simulink model "os_polyphase_synth_4096c"  (R2021a) (continued)
== system os_polyphase_synth_4096c/pfb_fir/pol1_in1_tap80 == (continued)
      | (D-ACIN)*(D-ACIN)+PCIN>>17+C+CARRYIN
      | (D-ACIN)*(D-ACIN)+PCIN>>17+CARRYIN
      | (D-ACIN)*(D-ACIN)+PCIN>>17+P
      | (D-ACIN)*(D-ACIN)+PCIN>>17+P+CARRYIN
      | (D-ACIN)*(D-ACIN)-C
      | (D-ACIN)*(D-ACIN)-P
      | (D-ACIN)*(D-ACIN)-P>>17
      | (D-ACIN)*(D-ACIN)-PCIN
      | (D-ACIN)*(D-ACIN)-PCIN>>17
      | (D-ACIN)*B
      | (D-ACIN)*B+C
      | (D-ACIN)*B+C+C
      | (D-ACIN)*B+C+C+CARRYIN
      | (D-ACIN)*B+C+CARRYIN
      | (D-ACIN)*B+C+P
      | (D-ACIN)*B+C+P+CARRYIN
      | (D-ACIN)*B+C+P>>17
      | (D-ACIN)*B+C+P>>17+CARRYIN
      | (D-ACIN)*B+C+PCIN
      | (D-ACIN)*B+C+PCIN+CARRYIN
      | (D-ACIN)*B+C+PCIN>>17
      | (D-ACIN)*B+C+PCIN>>17+CARRYIN
      | (D-ACIN)*B+C-C
      | (D-ACIN)*B+C-P
      | (D-ACIN)*B+C-P>>17
      | (D-ACIN)*B+C-PCIN
      | (D-ACIN)*B+C-PCIN>>17
      | (D-ACIN)*B+CARRYIN
      | (D-ACIN)*B+P
      | (D-ACIN)*B+P+C
      | (D-ACIN)*B+P+C+CARRYIN
      | (D-ACIN)*B+P+CARRYIN
      | (D-ACIN)*B+P+P
      | (D-ACIN)*B+P+P+CARRYIN
      | (D-ACIN)*B+P+P>>17
      | (D-ACIN)*B+P+P>>17+CARRYIN
      | (D-ACIN)*B+P+PCIN
      | (D-ACIN)*B+P+PCIN+CARRYIN
      | (D-ACIN)*B+P+PCIN>>17
      | (D-ACIN)*B+P+PCIN>>17+CARRYIN
      | (D-ACIN)*B+P-C
      | (D-ACIN)*B+P-P
      | (D-ACIN)*B+P-P>>17
      | (D-ACIN)*B+P-PCIN
      | (D-ACIN)*B+P-PCIN>>17
      | (D-ACIN)*B+P>>17
      | (D-ACIN)*B+P>>17+C
      | (D-ACIN)*B+P>>17+C+CARRYIN
      | (D-ACIN)*B+P>>17+CARRYIN
      | (D-ACIN)*B+P>>17+P
      | (D-ACIN)*B+P>>17+P+CARRYIN
      | (D-ACIN)*B+PCIN
      | (D-ACIN)*B+PCIN+C
      | (D-ACIN)*B+PCIN+C+CARRYIN
      | (D-ACIN)*B+PCIN+CARRYIN
      | (D-ACIN)*B+PCIN+P
      | (D-ACIN)*B+PCIN+P+CARRYIN
      | (D-ACIN)*B+PCIN>>17
      | (D-ACIN)*B+PCIN>>17+C
      | (D-ACIN)*B+PCIN>>17+C+CARRYIN
      | (D-ACIN)*B+PCIN>>17+CARRYIN
      | (D-ACIN)*B+PCIN>>17+P
      | (D-ACIN)*B+PCIN>>17+P+CARRYIN
      | (D-ACIN)*B-C
      | (D-ACIN)*B-P
      | (D-ACIN)*B-P>>17
      | (D-ACIN)*B-PCIN
      | (D-ACIN)*B-PCIN>>17
      | (D-ACIN)*BCIN
      | (D-ACIN)*BCIN+C
      | (D-ACIN)*BCIN+C+C
      | (D-ACIN)*BCIN+C+C+CARRYIN
      | (D-ACIN)*BCIN+C+CARRYIN
      | (D-ACIN)*BCIN+C+P
      | (D-ACIN)*BCIN+C+P+CARRYIN
      | (D-ACIN)*BCIN+C+P>>17
      | (D-ACIN)*BCIN+C+P>>17+CARRYIN
      | (D-ACIN)*BCIN+C+PCIN
      | (D-ACIN)*BCIN+C+PCIN+CARRYIN
      | (D-ACIN)*BCIN+C+PCIN>>17
      | (D-ACIN)*BCIN+C+PCIN>>17+CARRYIN
      | (D-ACIN)*BCIN+C-C
      | (D-ACIN)*BCIN+C-P
      | (D-ACIN)*BCIN+C-P>>17
      | (D-ACIN)*BCIN+C-PCIN
      | (D-ACIN)*BCIN+C-PCIN>>17
      | (D-ACIN)*BCIN+CARRYIN
      | (D-ACIN)*BCIN+P
      | (D-ACIN)*BCIN+P+C
      | (D-ACIN)*BCIN+P+C+CARRYIN
      | (D-ACIN)*BCIN+P+CARRYIN
      | (D-ACIN)*BCIN+P+P
      | (D-ACIN)*BCIN+P+P+CARRYIN
      | (D-ACIN)*BCIN+P+P>>17
      | (D-ACIN)*BCIN+P+P>>17+CARRYIN
      | (D-ACIN)*BCIN+P+PCIN
      | (D-ACIN)*BCIN+P+PCIN+CARRYIN
      | (D-ACIN)*BCIN+P+PCIN>>17
      | (D-ACIN)*BCIN+P+PCIN>>17+CARRYIN
      | (D-ACIN)*BCIN+P-C
      | (D-ACIN)*BCIN+P-P
      | (D-ACIN)*BCIN+P-P>>17
      | (D-ACIN)*BCIN+P-PCIN
      | (D-ACIN)*BCIN+P-PCIN>>17
      | (D-ACIN)*BCIN+P>>17
      | (D-ACIN)*BCIN+P>>17+C
      | (D-ACIN)*BCIN+P>>17+C+CARRYIN
      | (D-ACIN)*BCIN+P>>17+CARRYIN
      | (D-ACIN)*BCIN+P>>17+P
      | (D-ACIN)*BCIN+P>>17+P+CARRYIN
      | (D-ACIN)*BCIN+PCIN
      | (D-ACIN)*BCIN+PCIN+C
      | (D-ACIN)*BCIN+PCIN+C+CARRYIN
      | (D-ACIN)*BCIN+PCIN+CARRYIN
      | (D-ACIN)*BCIN+PCIN+P
      | (D-ACIN)*BCIN+PCIN+P+CARRYIN
      | (D-ACIN)*BCIN+PCIN>>17
      | (D-ACIN)*BCIN+PCIN>>17+C
      | (D-ACIN)*BCIN+PCIN>>17+C+CARRYIN
      | (D-ACIN)*BCIN+PCIN>>17+CARRYIN
      | (D-ACIN)*BCIN+PCIN>>17+P
      | (D-ACIN)*BCIN+PCIN>>17+P+CARRYIN
      | (D-ACIN)*BCIN-C
      | (D-ACIN)*BCIN-P
      | (D-ACIN)*BCIN-P>>17
      | (D-ACIN)*BCIN-PCIN
      | (D-ACIN)*BCIN-PCIN>>17
      | (D-ACIN)+C
      | (D-ACIN)+C+C
      | (D-ACIN)+C+C+CARRYIN
      | (D-ACIN)+C+CARRYIN
      | (D-ACIN)+C+P
      | (D-ACIN)+C+P+CARRYIN
      | (D-ACIN)+C+P>>17
      | (D-ACIN)+C+P>>17+CARRYIN
      | (D-ACIN)+C+PCIN
      | (D-ACIN)+C+PCIN+CARRYIN
      | (D-ACIN)+C+PCIN>>17
      | (D-ACIN)+C+PCIN>>17+CARRYIN
      | (D-ACIN)+C-C
      | (D-ACIN)+C-P
      | (D-ACIN)+C-P>>17
      | (D-ACIN)+C-PCIN
      | (D-ACIN)+C-PCIN>>17
      | (D-ACIN)+CARRYIN
      | (D-ACIN)+P
      | (D-ACIN)+P+C
      | (D-ACIN)+P+C+CARRYIN
      | (D-ACIN)+P+CARRYIN
      | (D-ACIN)+P+P
      | (D-ACIN)+P+P+CARRYIN
      | (D-ACIN)+P+P>>17
      | (D-ACIN)+P+P>>17+CARRYIN
      | (D-ACIN)+P+PCIN
      | (D-ACIN)+P+PCIN+CARRYIN
      | (D-ACIN)+P+PCIN>>17
      | (D-ACIN)+P+PCIN>>17+CARRYIN
      | (D-ACIN)+P-C
      | (D-ACIN)+P-P
      | (D-ACIN)+P-P>>17
      | (D-ACIN)+P-PCIN
      | (D-ACIN)+P-PCIN>>17
      | (D-ACIN)+P>>17
      | (D-ACIN)+P>>17+C
      | (D-ACIN)+P>>17+C+CARRYIN
      | (D-ACIN)+P>>17+CARRYIN
      | (D-ACIN)+P>>17+P
      | (D-ACIN)+P>>17+P+CARRYIN
      | (D-ACIN)+PCIN
      | (D-ACIN)+PCIN+C
      | (D-ACIN)+PCIN+C+CARRYIN
      | (D-ACIN)+PCIN+CARRYIN
      | (D-ACIN)+PCIN+P
      | (D-ACIN)+PCIN+P+CARRYIN
      | (D-ACIN)+PCIN>>17
      | (D-ACIN)+PCIN>>17+C
      | (D-ACIN)+PCIN>>17+C+CARRYIN
      | (D-ACIN)+PCIN>>17+CARRYIN
      | (D-ACIN)+PCIN>>17+P
      | (D-ACIN)+PCIN>>17+P+CARRYIN
      | (D-ACIN)-C
      | (D-ACIN)-P
      | (D-ACIN)-P>>17
      | (D-ACIN)-PCIN
      | (D-ACIN)-PCIN>>17
      | -((A+D))
      | -((A+D)*(A+D))
      | -((A+D)*(A+D)+C)
      | -((A+D)*(A+D)+C+CARRYIN)
      | -((A+D)*(A+D)+CARRYIN)
      | -((A+D)*(A+D)+P)
      | -((A+D)*(A+D)+P+CARRYIN)
      | -((A+D)*B)
      | -((A+D)*B+C)
      | -((A+D)*B+C+CARRYIN)
      | -((A+D)*B+CARRYIN)
      | -((A+D)*B+P)
      | -((A+D)*B+P+CARRYIN)
      | -((A+D)*BCIN)
      | -((A+D)*BCIN+C)
      | -((A+D)*BCIN+C+CARRYIN)
      | -((A+D)*BCIN+CARRYIN)
      | -((A+D)*BCIN+P)
      | -((A+D)*BCIN+P+CARRYIN)
      | -((A+D)+C)
      | -((A+D)+C+CARRYIN)
      | -((A+D)+CARRYIN)
      | -((A+D)+P)
      | -((A+D)+P+CARRYIN)
      | -((ACIN+D))
      | -((ACIN+D)*(ACIN+D))
      | -((ACIN+D)*(ACIN+D)+C)
      | -((ACIN+D)*(ACIN+D)+C+CARRYIN)
      | -((ACIN+D)*(ACIN+D)+CARRYIN)
      | -((ACIN+D)*(ACIN+D)+P)
      | -((ACIN+D)*(ACIN+D)+P+CARRYIN)
      | -((ACIN+D)*B)
      | -((ACIN+D)*B+C)
      | -((ACIN+D)*B+C+CARRYIN)
      | -((ACIN+D)*B+CARRYIN)
      | -((ACIN+D)*B+P)
      | -((ACIN+D)*B+P+CARRYIN)
      | -((ACIN+D)*BCIN)
      | -((ACIN+D)*BCIN+C)
      | -((ACIN+D)*BCIN+C+CARRYIN)
      | -((ACIN+D)*BCIN+CARRYIN)
      | -((ACIN+D)*BCIN+P)
      | -((ACIN+D)*BCIN+P+CARRYIN)
      | -((ACIN+D)+C)
      | -((ACIN+D)+C+CARRYIN)
      | -((ACIN+D)+CARRYIN)
      | -((ACIN+D)+P)
      | -((ACIN+D)+P+CARRYIN)
      | -((D+A))
      | -((D+A)*(D+A))
      | -((D+A)*(D+A)+C)
      | -((D+A)*(D+A)+C+CARRYIN)
      | -((D+A)*(D+A)+CARRYIN)
      | -((D+A)*(D+A)+P)
      | -((D+A)*(D+A)+P+CARRYIN)
      | -((D+A)*B)
      | -((D+A)*B+C)
      | -((D+A)*B+C+CARRYIN)
      | -((D+A)*B+CARRYIN)
      | -((D+A)*B+P)
      | -((D+A)*B+P+CARRYIN)
      | -((D+A)*BCIN)
      | -((D+A)*BCIN+C)
      | -((D+A)*BCIN+C+CARRYIN)
      | -((D+A)*BCIN+CARRYIN)
      | -((D+A)*BCIN+P)
      | -((D+A)*BCIN+P+CARRYIN)
      | -((D+A)+C)
      | -((D+A)+C+CARRYIN)
      | -((D+A)+CARRYIN)
      | -((D+A)+P)
      | -((D+A)+P+CARRYIN)
      | -((D+ACIN))
      | -((D+ACIN)*(D+ACIN))
      | -((D+ACIN)*(D+ACIN)+C)
      | -((D+ACIN)*(D+ACIN)+C+CARRYIN)
      | -((D+ACIN)*(D+ACIN)+CARRYIN)
      | -((D+ACIN)*(D+ACIN)+P)
      | -((D+ACIN)*(D+ACIN)+P+CARRYIN)
      | -((D+ACIN)*B)
      | -((D+ACIN)*B+C)
      | -((D+ACIN)*B+C+CARRYIN)
      | -((D+ACIN)*B+CARRYIN)
      | -((D+ACIN)*B+P)
      | -((D+ACIN)*B+P+CARRYIN)
      | -((D+ACIN)*BCIN)
      | -((D+ACIN)*BCIN+C)
      | -((D+ACIN)*BCIN+C+CARRYIN)
      | -((D+ACIN)*BCIN+CARRYIN)
      | -((D+ACIN)*BCIN+P)
      | -((D+ACIN)*BCIN+P+CARRYIN)
      | -((D+ACIN)+C)
      | -((D+ACIN)+C+CARRYIN)
      | -((D+ACIN)+CARRYIN)
      | -((D+ACIN)+P)
      | -((D+ACIN)+P+CARRYIN)
      | -((D-A))
      | -((D-A)*(D-A))
      | -((D-A)*(D-A)+C)
      | -((D-A)*(D-A)+C+CARRYIN)
      | -((D-A)*(D-A)+CARRYIN)
      | -((D-A)*(D-A)+P)
      | -((D-A)*(D-A)+P+CARRYIN)
      | -((D-A)*B)
      | -((D-A)*B+C)
      | -((D-A)*B+C+CARRYIN)
      | -((D-A)*B+CARRYIN)
      | -((D-A)*B+P)
      | -((D-A)*B+P+CARRYIN)
      | -((D-A)*BCIN)
      | -((D-A)*BCIN+C)
      | -((D-A)*BCIN+C+CARRYIN)
      | -((D-A)*BCIN+CARRYIN)
      | -((D-A)*BCIN+P)
      | -((D-A)*BCIN+P+CARRYIN)
      | -((D-A)+C)
      | -((D-A)+C+CARRYIN)
      | -((D-A)+CARRYIN)
      | -((D-A)+P)
      | -((D-A)+P+CARRYIN)
      | -((D-ACIN))
      | -((D-ACIN)*(D-ACIN))
      | -((D-ACIN)*(D-ACIN)+C)
      | -((D-ACIN)*(D-ACIN)+C+CARRYIN)
      | -((D-ACIN)*(D-ACIN)+CARRYIN)
      | -((D-ACIN)*(D-ACIN)+P)
      | -((D-ACIN)*(D-ACIN)+P+CARRYIN)
      | -((D-ACIN)*B)
      | -((D-ACIN)*B+C)
      | -((D-ACIN)*B+C+CARRYIN)
      | -((D-ACIN)*B+CARRYIN)
      | -((D-ACIN)*B+P)
      | -((D-ACIN)*B+P+CARRYIN)
      | -((D-ACIN)*BCIN)
      | -((D-ACIN)*BCIN+C)
      | -((D-ACIN)*BCIN+C+CARRYIN)
      | -((D-ACIN)*BCIN+CARRYIN)
      | -((D-ACIN)*BCIN+P)
      | -((D-ACIN)*BCIN+P+CARRYIN)
      | -((D-ACIN)+C)
      | -((D-ACIN)+C+CARRYIN)
      | -((D-ACIN)+CARRYIN)
      | -((D-ACIN)+P)
      | -((D-ACIN)+P+CARRYIN)
      | -(A)
      | -(A*A)
      | -(A*A+C)
      | -(A*A+C+CARRYIN)
      | -(A*A+CARRYIN)
      | -(A*A+P)
      | -(A*A+P+CARRYIN)
      | -(A*B)
      | -(A*B+C)
      | -(A*B+C+CARRYIN)
      | -(A*B+CARRYIN)
      | -(A*B+P)
      | -(A*B+P+CARRYIN)
      | -(A*BCIN)
      | -(A*BCIN+C)
      | -(A*BCIN+C+CARRYIN)
      | -(A*BCIN+CARRYIN)
      | -(A*BCIN+P)
      | -(A*BCIN+P+CARRYIN)
      | -(A+C)
      | -(A+C+CARRYIN)
      | -(A+CARRYIN)
      | -(A+D)
      | -(A+D)*(A+D)
      | -(A+D)*(A+D)-C
      | -(A+D)*(A+D)-C-CARRYIN
      | -(A+D)*(A+D)-CARRYIN
      | -(A+D)*(A+D)-P
      | -(A+D)*(A+D)-P-CARRYIN
      | -(A+D)*B
      | -(A+D)*B-C
      | -(A+D)*B-C-CARRYIN
      | -(A+D)*B-CARRYIN
      | -(A+D)*B-P
      | -(A+D)*B-P-CARRYIN
      | -(A+D)*BCIN
      | -(A+D)*BCIN-C
      | -(A+D)*BCIN-C-CARRYIN
      | -(A+D)*BCIN-CARRYIN
      | -(A+D)*BCIN-P
      | -(A+D)*BCIN-P-CARRYIN
      | -(A+D)-C
      | -(A+D)-C-CARRYIN
      | -(A+D)-CARRYIN
      | -(A+D)-P
      | -(A+D)-P-CARRYIN
      | -(A+P)
      | -(A+P+CARRYIN)
      | -(ACIN)
      | -(ACIN*ACIN)
      | -(ACIN*ACIN+C)
      | -(ACIN*ACIN+C+CARRYIN)
      | -(ACIN*ACIN+CARRYIN)
      | -(ACIN*ACIN+P)
      | -(ACIN*ACIN+P+CARRYIN)
      | -(ACIN*B)
      | -(ACIN*B+C)
      | -(ACIN*B+C+CARRYIN)
      | -(ACIN*B+CARRYIN)
      | -(ACIN*B+P)
      | -(ACIN*B+P+CARRYIN)
      | -(ACIN*BCIN)
      | -(ACIN*BCIN+C)
      | -(ACIN*BCIN+C+CARRYIN)
      | -(ACIN*BCIN+CARRYIN)
      | -(ACIN*BCIN+P)
      | -(ACIN*BCIN+P+CARRYIN)
      | -(ACIN+C)
      | -(ACIN+C+CARRYIN)
      | -(ACIN+CARRYIN)
      | -(ACIN+D)
      | -(ACIN+D)*(ACIN+D)
      | -(ACIN+D)*(ACIN+D)-C
      | -(ACIN+D)*(ACIN+D)-C-CARRYIN
      | -(ACIN+D)*(ACIN+D)-CARRYIN
      | -(ACIN+D)*(ACIN+D)-P
      | -(ACIN+D)*(ACIN+D)-P-CARRYIN
      | -(ACIN+D)*B
      | -(ACIN+D)*B-C
      | -(ACIN+D)*B-C-CARRYIN
      | -(ACIN+D)*B-CARRYIN
      | -(ACIN+D)*B-P
      | -(ACIN+D)*B-P-CARRYIN
      | -(ACIN+D)*BCIN
      | -(ACIN+D)*BCIN-C
      | -(ACIN+D)*BCIN-C-CARRYIN
      | -(ACIN+D)*BCIN-CARRYIN
      | -(ACIN+D)*BCIN-P
      | -(ACIN+D)*BCIN-P-CARRYIN
      | -(ACIN+D)-C
      | -(ACIN+D)-C-CARRYIN
      | -(ACIN+D)-CARRYIN
      | -(ACIN+D)-P
      | -(ACIN+D)-P-CARRYIN
      | -(ACIN+P)
      | -(ACIN+P+CARRYIN)
      | -(B*(A+D))
      | -(B*(A+D)+C)
      | -(B*(A+D)+C+CARRYIN)
      | -(B*(A+D)+CARRYIN)
      | -(B*(A+D)+P)
      | -(B*(A+D)+P+CARRYIN)
      | -(B*(ACIN+D))
      | -(B*(ACIN+D)+C)
      | -(B*(ACIN+D)+C+CARRYIN)
      | -(B*(ACIN+D)+CARRYIN)
      | -(B*(ACIN+D)+P)
      | -(B*(ACIN+D)+P+CARRYIN)
      | -(B*(D+A))
      | -(B*(D+A)+C)
      | -(B*(D+A)+C+CARRYIN)
      | -(B*(D+A)+CARRYIN)
      | -(B*(D+A)+P)
      | -(B*(D+A)+P+CARRYIN)
      | -(B*(D+ACIN))
      | -(B*(D+ACIN)+C)
      | -(B*(D+ACIN)+C+CARRYIN)
      | -(B*(D+ACIN)+CARRYIN)
      | -(B*(D+ACIN)+P)
      | -(B*(D+ACIN)+P+CARRYIN)
      | -(B*(D-A))
      | -(B*(D-A)+C)
      | -(B*(D-A)+C+CARRYIN)
      | -(B*(D-A)+CARRYIN)
      | -(B*(D-A)+P)
      | -(B*(D-A)+P+CARRYIN)
      | -(B*(D-ACIN))
      | -(B*(D-ACIN)+C)
      | -(B*(D-ACIN)+C+CARRYIN)
      | -(B*(D-ACIN)+CARRYIN)
      | -(B*(D-ACIN)+P)
      | -(B*(D-ACIN)+P+CARRYIN)
      | -(B*A)
      | -(B*A+C)
      | -(B*A+C+CARRYIN)
      | -(B*A+CARRYIN)
      | -(B*A+P)
      | -(B*A+P+CARRYIN)
      | -(B*ACIN)
      | -(B*ACIN+C)
      | -(B*ACIN+C+CARRYIN)
      | -(B*ACIN+CARRYIN)
      | -(B*ACIN+P)
      | -(B*ACIN+P+CARRYIN)
      | -(B*D)
      | -(B*D+C)
      | -(B*D+C+CARRYIN)
      | -(B*D+CARRYIN)
      | -(B*D+P)
      | -(B*D+P+CARRYIN)
      | -(BCIN*(A+D))
      | -(BCIN*(A+D)+C)
      | -(BCIN*(A+D)+C+CARRYIN)
      | -(BCIN*(A+D)+CARRYIN)
      | -(BCIN*(A+D)+P)
      | -(BCIN*(A+D)+P+CARRYIN)
      | -(BCIN*(ACIN+D))
      | -(BCIN*(ACIN+D)+C)
      | -(BCIN*(ACIN+D)+C+CARRYIN)
      | -(BCIN*(ACIN+D)+CARRYIN)
      | -(BCIN*(ACIN+D)+P)
      | -(BCIN*(ACIN+D)+P+CARRYIN)
      | -(BCIN*(D+A))
      | -(BCIN*(D+A)+C)
      | -(BCIN*(D+A)+C+CARRYIN)
      | -(BCIN*(D+A)+CARRYIN)
      | -(BCIN*(D+A)+P)
      | -(BCIN*(D+A)+P+CARRYIN)
      | -(BCIN*(D+ACIN))
      | -(BCIN*(D+ACIN)+C)
      | -(BCIN*(D+ACIN)+C+CARRYIN)
      | -(BCIN*(D+ACIN)+CARRYIN)
      | -(BCIN*(D+ACIN)+P)
      | -(BCIN*(D+ACIN)+P+CARRYIN)
      | -(BCIN*(D-A))
      | -(BCIN*(D-A)+C)
      | -(BCIN*(D-A)+C+CARRYIN)
      | -(BCIN*(D-A)+CARRYIN)
      | -(BCIN*(D-A)+P)
      | -(BCIN*(D-A)+P+CARRYIN)
      | -(BCIN*(D-ACIN))
      | -(BCIN*(D-ACIN)+C)
      | -(BCIN*(D-ACIN)+C+CARRYIN)
      | -(BCIN*(D-ACIN)+CARRYIN)
      | -(BCIN*(D-ACIN)+P)
      | -(BCIN*(D-ACIN)+P+CARRYIN)
      | -(BCIN*A)
      | -(BCIN*A+C)
      | -(BCIN*A+C+CARRYIN)
      | -(BCIN*A+CARRYIN)
      | -(BCIN*A+P)
      | -(BCIN*A+P+CARRYIN)
      | -(BCIN*ACIN)
      | -(BCIN*ACIN+C)
      | -(BCIN*ACIN+C+CARRYIN)
      | -(BCIN*ACIN+CARRYIN)
      | -(BCIN*ACIN+P)
      | -(BCIN*ACIN+P+CARRYIN)
      | -(BCIN*D)
      | -(BCIN*D+C)
      | -(BCIN*D+C+CARRYIN)
      | -(BCIN*D+CARRYIN)
      | -(BCIN*D+P)
      | -(BCIN*D+P+CARRYIN)
      | -(C)
      | -(C+(A+D))
      | -(C+(A+D)*(A+D))
      | -(C+(A+D)*(A+D)+CARRYIN)
      | -(C+(A+D)*B)
      | -(C+(A+D)*B+CARRYIN)
      | -(C+(A+D)*BCIN)
      | -(C+(A+D)*BCIN+CARRYIN)
      | -(C+(A+D)+CARRYIN)
      | -(C+(ACIN+D))
      | -(C+(ACIN+D)*(ACIN+D))
      | -(C+(ACIN+D)*(ACIN+D)+CARRYIN)
      | -(C+(ACIN+D)*B)
      | -(C+(ACIN+D)*B+CARRYIN)
      | -(C+(ACIN+D)*BCIN)
      | -(C+(ACIN+D)*BCIN+CARRYIN)
      | -(C+(ACIN+D)+CARRYIN)
      | -(C+(D+A))
      | -(C+(D+A)*(D+A))
      | -(C+(D+A)*(D+A)+CARRYIN)
      | -(C+(D+A)*B)
      | -(C+(D+A)*B+CARRYIN)
      | -(C+(D+A)*BCIN)
      | -(C+(D+A)*BCIN+CARRYIN)
      | -(C+(D+A)+CARRYIN)
      | -(C+(D+ACIN))
      | -(C+(D+ACIN)*(D+ACIN))
      | -(C+(D+ACIN)*(D+ACIN)+CARRYIN)
      | -(C+(D+ACIN)*B)
      | -(C+(D+ACIN)*B+CARRYIN)
      | -(C+(D+ACIN)*BCIN)
      | -(C+(D+ACIN)*BCIN+CARRYIN)
      | -(C+(D+ACIN)+CARRYIN)
      | -(C+(D-A))
      | -(C+(D-A)*(D-A))
      | -(C+(D-A)*(D-A)+CARRYIN)
      | -(C+(D-A)*B)
      | -(C+(D-A)*B+CARRYIN)
      | -(C+(D-A)*BCIN)
      | -(C+(D-A)*BCIN+CARRYIN)
      | -(C+(D-A)+CARRYIN)
      | -(C+(D-ACIN))
      | -(C+(D-ACIN)*(D-ACIN))
      | -(C+(D-ACIN)*(D-ACIN)+CARRYIN)
      | -(C+(D-ACIN)*B)
      | -(C+(D-ACIN)*B+CARRYIN)
      | -(C+(D-ACIN)*BCIN)
      | -(C+(D-ACIN)*BCIN+CARRYIN)
      | -(C+(D-ACIN)+CARRYIN)
      | -(C+A)
      | -(C+A*A)
      | -(C+A*A+CARRYIN)
      | -(C+A*B)
      | -(C+A*B+CARRYIN)
      | -(C+A*BCIN)
      | -(C+A*BCIN+CARRYIN)
      | -(C+A+CARRYIN)
      | -(C+ACIN)
      | -(C+ACIN*ACIN)
      | -(C+ACIN*ACIN+CARRYIN)
      | -(C+ACIN*B)
      | -(C+ACIN*B+CARRYIN)
      | -(C+ACIN*BCIN)
      | -(C+ACIN*BCIN+CARRYIN)
      | -(C+ACIN+CARRYIN)
      | -(C+B*(A+D))
      | -(C+B*(A+D)+CARRYIN)
      | -(C+B*(ACIN+D))
      | -(C+B*(ACIN+D)+CARRYIN)
      | -(C+B*(D+A))
      | -(C+B*(D+A)+CARRYIN)
      | -(C+B*(D+ACIN))
      | -(C+B*(D+ACIN)+CARRYIN)
      | -(C+B*(D-A))
      | -(C+B*(D-A)+CARRYIN)
      | -(C+B*(D-ACIN))
      | -(C+B*(D-ACIN)+CARRYIN)
      | -(C+B*A)
      | -(C+B*A+CARRYIN)
      | -(C+B*ACIN)
      | -(C+B*ACIN+CARRYIN)
      | -(C+B*D)
      | -(C+B*D+CARRYIN)
      | -(C+BCIN*(A+D))
      | -(C+BCIN*(A+D)+CARRYIN)
      | -(C+BCIN*(ACIN+D))
      | -(C+BCIN*(ACIN+D)+CARRYIN)
      | -(C+BCIN*(D+A))
      | -(C+BCIN*(D+A)+CARRYIN)
      | -(C+BCIN*(D+ACIN))
      | -(C+BCIN*(D+ACIN)+CARRYIN)
      | -(C+BCIN*(D-A))
      | -(C+BCIN*(D-A)+CARRYIN)
      | -(C+BCIN*(D-ACIN))
      | -(C+BCIN*(D-ACIN)+CARRYIN)
      | -(C+BCIN*A)
      | -(C+BCIN*A+CARRYIN)
      | -(C+BCIN*ACIN)
      | -(C+BCIN*ACIN+CARRYIN)
      | -(C+BCIN*D)
      | -(C+BCIN*D+CARRYIN)
      | -(C+C)
      | -(C+C+CARRYCASCIN)
      | -(C+C+CARRYIN)
      | -(C+C+CONCAT)
      | -(C+C+CONCAT+CARRYIN)
      | -(C+C+P)
      | -(C+C+P+CARRYIN)
      | -(C+CARRYCASCIN)
      | -(C+CARRYIN)
      | -(C+CONCAT)
      | -(C+CONCAT+C)
      | -(C+CONCAT+C+CARRYIN)
      | -(C+CONCAT+CARRYCASCIN)
      | -(C+CONCAT+CARRYIN)
      | -(C+CONCAT+P)
      | -(C+CONCAT+P+CARRYIN)
      | -(C+D)
      | -(C+D*B)
      | -(C+D*B+CARRYIN)
      | -(C+D*BCIN)
      | -(C+D*BCIN+CARRYIN)
      | -(C+D*D)
      | -(C+D*D+CARRYIN)
      | -(C+D+CARRYIN)
      | -(C+P)
      | -(C+P+C)
      | -(C+P+C+CARRYIN)
      | -(C+P+CARRYCASCIN)
      | -(C+P+CARRYIN)
      | -(C+P+CONCAT)
      | -(C+P+CONCAT+CARRYIN)
      | -(C+P+P)
      | -(C+P+P+CARRYIN)
      | -(CARRYIN)
      | -(CONCAT)
      | -(CONCAT+C)
      | -(CONCAT+C+C)
      | -(CONCAT+C+C+CARRYIN)
      | -(CONCAT+C+CARRYCASCIN)
      | -(CONCAT+C+CARRYIN)
      | -(CONCAT+C+P)
      | -(CONCAT+C+P+CARRYIN)
      | -(CONCAT+CARRYCASCIN)
      | -(CONCAT+CARRYIN)
      | -(CONCAT+P)
      | -(CONCAT+P+C)
      | -(CONCAT+P+C+CARRYIN)
      | -(CONCAT+P+CARRYCASCIN)
      | -(CONCAT+P+CARRYIN)
      | -(D)
      | -(D*B)
      | -(D*B+C)
      | -(D*B+C+CARRYIN)
      | -(D*B+CARRYIN)
      | -(D*B+P)
      | -(D*B+P+CARRYIN)
      | -(D*BCIN)
      | -(D*BCIN+C)
      | -(D*BCIN+C+CARRYIN)
      | -(D*BCIN+CARRYIN)
      | -(D*BCIN+P)
      | -(D*BCIN+P+CARRYIN)
      | -(D*D)
      | -(D*D+C)
      | -(D*D+C+CARRYIN)
      | -(D*D+CARRYIN)
      | -(D*D+P)
      | -(D*D+P+CARRYIN)
      | -(D+A)
      | -(D+A)*(D+A)
      | -(D+A)*(D+A)-C
      | -(D+A)*(D+A)-C-CARRYIN
      | -(D+A)*(D+A)-CARRYIN
      | -(D+A)*(D+A)-P
      | -(D+A)*(D+A)-P-CARRYIN
      | -(D+A)*B
      | -(D+A)*B-C
      | -(D+A)*B-C-CARRYIN
      | -(D+A)*B-CARRYIN
      | -(D+A)*B-P
      | -(D+A)*B-P-CARRYIN
      | -(D+A)*BCIN
      | -(D+A)*BCIN-C
      | -(D+A)*BCIN-C-CARRYIN
      | -(D+A)*BCIN-CARRYIN
      | -(D+A)*BCIN-P
      | -(D+A)*BCIN-P-CARRYIN
      | -(D+A)-C
      | -(D+A)-C-CARRYIN
      | -(D+A)-CARRYIN
      | -(D+A)-P
      | -(D+A)-P-CARRYIN
      | -(D+ACIN)
      | -(D+ACIN)*(D+ACIN)
      | -(D+ACIN)*(D+ACIN)-C
      | -(D+ACIN)*(D+ACIN)-C-CARRYIN
      | -(D+ACIN)*(D+ACIN)-CARRYIN
      | -(D+ACIN)*(D+ACIN)-P
      | -(D+ACIN)*(D+ACIN)-P-CARRYIN
      | -(D+ACIN)*B
      | -(D+ACIN)*B-C
      | -(D+ACIN)*B-C-CARRYIN
      | -(D+ACIN)*B-CARRYIN
      | -(D+ACIN)*B-P
      | -(D+ACIN)*B-P-CARRYIN
      | -(D+ACIN)*BCIN
      | -(D+ACIN)*BCIN-C
      | -(D+ACIN)*BCIN-C-CARRYIN
      | -(D+ACIN)*BCIN-CARRYIN
      | -(D+ACIN)*BCIN-P
      | -(D+ACIN)*BCIN-P-CARRYIN
      | -(D+ACIN)-C
      | -(D+ACIN)-C-CARRYIN
      | -(D+ACIN)-CARRYIN
      | -(D+ACIN)-P
      | -(D+ACIN)-P-CARRYIN
      | -(D+C)
      | -(D+C+CARRYIN)
      | -(D+CARRYIN)
      | -(D+P)
      | -(D+P+CARRYIN)
      | -(D-A)
      | -(D-A)*(D-A)
      | -(D-A)*(D-A)-C
      | -(D-A)*(D-A)-C-CARRYIN
      | -(D-A)*(D-A)-CARRYIN
      | -(D-A)*(D-A)-P
      | -(D-A)*(D-A)-P-CARRYIN
      | -(D-A)*B
      | -(D-A)*B-C
      | -(D-A)*B-C-CARRYIN
      | -(D-A)*B-CARRYIN
      | -(D-A)*B-P
      | -(D-A)*B-P-CARRYIN
      | -(D-A)*BCIN
      | -(D-A)*BCIN-C
      | -(D-A)*BCIN-C-CARRYIN
      | -(D-A)*BCIN-CARRYIN
      | -(D-A)*BCIN-P
      | -(D-A)*BCIN-P-CARRYIN
      | -(D-A)-C
      | -(D-A)-C-CARRYIN
      | -(D-A)-CARRYIN
      | -(D-A)-P
      | -(D-A)-P-CARRYIN
      | -(D-ACIN)
      | -(D-ACIN)*(D-ACIN)
      | -(D-ACIN)*(D-ACIN)-C
      | -(D-ACIN)*(D-ACIN)-C-CARRYIN
      | -(D-ACIN)*(D-ACIN)-CARRYIN
      | -(D-ACIN)*(D-ACIN)-P
      | -(D-ACIN)*(D-ACIN)-P-CARRYIN
      | -(D-ACIN)*B
      | -(D-ACIN)*B-C
      | -(D-ACIN)*B-C-CARRYIN
      | -(D-ACIN)*B-CARRYIN
      | -(D-ACIN)*B-P
      | -(D-ACIN)*B-P-CARRYIN
      | -(D-ACIN)*BCIN
      | -(D-ACIN)*BCIN-C
      | -(D-ACIN)*BCIN-C-CARRYIN
      | -(D-ACIN)*BCIN-CARRYIN
      | -(D-ACIN)*BCIN-P
      | -(D-ACIN)*BCIN-P-CARRYIN
      | -(D-ACIN)-C
      | -(D-ACIN)-C-CARRYIN
      | -(D-ACIN)-CARRYIN
      | -(D-ACIN)-P
      | -(D-ACIN)-P-CARRYIN
      | -(P)
      | -(P+(A+D))
      | -(P+(A+D)*(A+D))
      | -(P+(A+D)*(A+D)+CARRYIN)
      | -(P+(A+D)*B)
      | -(P+(A+D)*B+CARRYIN)
      | -(P+(A+D)*BCIN)
      | -(P+(A+D)*BCIN+CARRYIN)
      | -(P+(A+D)+CARRYIN)
      | -(P+(ACIN+D))
      | -(P+(ACIN+D)*(ACIN+D))
      | -(P+(ACIN+D)*(ACIN+D)+CARRYIN)
      | -(P+(ACIN+D)*B)
      | -(P+(ACIN+D)*B+CARRYIN)
      | -(P+(ACIN+D)*BCIN)
      | -(P+(ACIN+D)*BCIN+CARRYIN)
      | -(P+(ACIN+D)+CARRYIN)
      | -(P+(D+A))
      | -(P+(D+A)*(D+A))
      | -(P+(D+A)*(D+A)+CARRYIN)
      | -(P+(D+A)*B)
      | -(P+(D+A)*B+CARRYIN)
      | -(P+(D+A)*BCIN)
      | -(P+(D+A)*BCIN+CARRYIN)
      | -(P+(D+A)+CARRYIN)
      | -(P+(D+ACIN))
      | -(P+(D+ACIN)*(D+ACIN))
      | -(P+(D+ACIN)*(D+ACIN)+CARRYIN)
      | -(P+(D+ACIN)*B)
      | -(P+(D+ACIN)*B+CARRYIN)
      | -(P+(D+ACIN)*BCIN)
      | -(P+(D+ACIN)*BCIN+CARRYIN)
      | -(P+(D+ACIN)+CARRYIN)
      | -(P+(D-A))
      | -(P+(D-A)*(D-A))
      | -(P+(D-A)*(D-A)+CARRYIN)
      | -(P+(D-A)*B)
      | -(P+(D-A)*B+CARRYIN)
      | -(P+(D-A)*BCIN)
      | -(P+(D-A)*BCIN+CARRYIN)
      | -(P+(D-A)+CARRYIN)
      | -(P+(D-ACIN))
      | -(P+(D-ACIN)*(D-ACIN))
      | -(P+(D-ACIN)*(D-ACIN)+CARRYIN)
      | -(P+(D-ACIN)*B)
      | -(P+(D-ACIN)*B+CARRYIN)
      | -(P+(D-ACIN)*BCIN)
      | -(P+(D-ACIN)*BCIN+CARRYIN)
      | -(P+(D-ACIN)+CARRYIN)
      | -(P+A)
      | -(P+A*A)
      | -(P+A*A+CARRYIN)
      | -(P+A*B)
      | -(P+A*B+CARRYIN)
      | -(P+A*BCIN)
      | -(P+A*BCIN+CARRYIN)
      | -(P+A+CARRYIN)
      | -(P+ACIN)
      | -(P+ACIN*ACIN)
      | -(P+ACIN*ACIN+CARRYIN)
      | -(P+ACIN*B)
      | -(P+ACIN*B+CARRYIN)
      | -(P+ACIN*BCIN)
      | -(P+ACIN*BCIN+CARRYIN)
      | -(P+ACIN+CARRYIN)
      | -(P+B*(A+D))
      | -(P+B*(A+D)+CARRYIN)
      | -(P+B*(ACIN+D))
      | -(P+B*(ACIN+D)+CARRYIN)
      | -(P+B*(D+A))
      | -(P+B*(D+A)+CARRYIN)
      | -(P+B*(D+ACIN))
      | -(P+B*(D+ACIN)+CARRYIN)
      | -(P+B*(D-A))
      | -(P+B*(D-A)+CARRYIN)
      | -(P+B*(D-ACIN))
      | -(P+B*(D-ACIN)+CARRYIN)
      | -(P+B*A)
      | -(P+B*A+CARRYIN)
      | -(P+B*ACIN)
      | -(P+B*ACIN+CARRYIN)
      | -(P+B*D)
      | -(P+B*D+CARRYIN)
      | -(P+BCIN*(A+D))
      | -(P+BCIN*(A+D)+CARRYIN)
      | -(P+BCIN*(ACIN+D))
      | -(P+BCIN*(ACIN+D)+CARRYIN)
      | -(P+BCIN*(D+A))
      | -(P+BCIN*(D+A)+CARRYIN)
      | -(P+BCIN*(D+ACIN))
      | -(P+BCIN*(D+ACIN)+CARRYIN)
      | -(P+BCIN*(D-A))
      | -(P+BCIN*(D-A)+CARRYIN)
      | -(P+BCIN*(D-ACIN))
      | -(P+BCIN*(D-ACIN)+CARRYIN)
      | -(P+BCIN*A)
      | -(P+BCIN*A+CARRYIN)
      | -(P+BCIN*ACIN)
      | -(P+BCIN*ACIN+CARRYIN)
      | -(P+BCIN*D)
      | -(P+BCIN*D+CARRYIN)
      | -(P+C)
      | -(P+C+C)
      | -(P+C+C+CARRYIN)
      | -(P+C+CARRYCASCIN)
      | -(P+C+CARRYIN)
      | -(P+C+CONCAT)
      | -(P+C+CONCAT+CARRYIN)
      | -(P+C+P)
      | -(P+C+P+CARRYIN)
      | -(P+CARRYCASCIN)
      | -(P+CARRYIN)
      | -(P+CONCAT)
      | -(P+CONCAT+C)
      | -(P+CONCAT+C+CARRYIN)
      | -(P+CONCAT+CARRYCASCIN)
      | -(P+CONCAT+CARRYIN)
      | -(P+D)
      | -(P+D*B)
      | -(P+D*B+CARRYIN)
      | -(P+D*BCIN)
      | -(P+D*BCIN+CARRYIN)
      | -(P+D*D)
      | -(P+D*D+CARRYIN)
      | -(P+D+CARRYIN)
      | -(P+P)
      | -(P+P+C)
      | -(P+P+C+CARRYIN)
      | -(P+P+CARRYCASCIN)
      | -(P+P+CARRYIN)
      | -A
      | -A*A
      | -A*A-C
      | -A*A-C-CARRYIN
      | -A*A-CARRYIN
      | -A*A-P
      | -A*A-P-CARRYIN
      | -A*B
      | -A*B-C
      | -A*B-C-CARRYIN
      | -A*B-CARRYIN
      | -A*B-P
      | -A*B-P-CARRYIN
      | -A*BCIN
      | -A*BCIN-C
      | -A*BCIN-C-CARRYIN
      | -A*BCIN-CARRYIN
      | -A*BCIN-P
      | -A*BCIN-P-CARRYIN
      | -A-C
      | -A-C-CARRYIN
      | -A-CARRYIN
      | -A-P
      | -A-P-CARRYIN
      | -ACIN
      | -ACIN*ACIN
      | -ACIN*ACIN-C
      | -ACIN*ACIN-C-CARRYIN
      | -ACIN*ACIN-CARRYIN
      | -ACIN*ACIN-P
      | -ACIN*ACIN-P-CARRYIN
      | -ACIN*B
      | -ACIN*B-C
      | -ACIN*B-C-CARRYIN
      | -ACIN*B-CARRYIN
      | -ACIN*B-P
      | -ACIN*B-P-CARRYIN
      | -ACIN*BCIN
      | -ACIN*BCIN-C
      | -ACIN*BCIN-C-CARRYIN
      | -ACIN*BCIN-CARRYIN
      | -ACIN*BCIN-P
      | -ACIN*BCIN-P-CARRYIN
      | -ACIN-C
      | -ACIN-C-CARRYIN
      | -ACIN-CARRYIN
      | -ACIN-P
      | -ACIN-P-CARRYIN
      | -B*(A+D)
      | -B*(A+D)-C
      | -B*(A+D)-C-CARRYIN
      | -B*(A+D)-CARRYIN
      | -B*(A+D)-P
      | -B*(A+D)-P-CARRYIN
      | -B*(ACIN+D)
      | -B*(ACIN+D)-C
      | -B*(ACIN+D)-C-CARRYIN
      | -B*(ACIN+D)-CARRYIN
      | -B*(ACIN+D)-P
      | -B*(ACIN+D)-P-CARRYIN
      | -B*(D+A)
      | -B*(D+A)-C
      | -B*(D+A)-C-CARRYIN
      | -B*(D+A)-CARRYIN
      | -B*(D+A)-P
      | -B*(D+A)-P-CARRYIN
      | -B*(D+ACIN)
      | -B*(D+ACIN)-C
      | -B*(D+ACIN)-C-CARRYIN
      | -B*(D+ACIN)-CARRYIN
      | -B*(D+ACIN)-P
      | -B*(D+ACIN)-P-CARRYIN
      | -B*(D-A)
      | -B*(D-A)-C
      | -B*(D-A)-C-CARRYIN
      | -B*(D-A)-CARRYIN
      | -B*(D-A)-P
      | -B*(D-A)-P-CARRYIN
      | -B*(D-ACIN)
      | -B*(D-ACIN)-C
      | -B*(D-ACIN)-C-CARRYIN
      | -B*(D-ACIN)-CARRYIN
      | -B*(D-ACIN)-P
      | -B*(D-ACIN)-P-CARRYIN
      | -B*A
      | -B*A-C
      | -B*A-C-CARRYIN
      | -B*A-CARRYIN
      | -B*A-P
      | -B*A-P-CARRYIN
      | -B*ACIN
      | -B*ACIN-C
      | -B*ACIN-C-CARRYIN
      | -B*ACIN-CARRYIN
      | -B*ACIN-P
      | -B*ACIN-P-CARRYIN
      | -B*D
      | -B*D-C
      | -B*D-C-CARRYIN
      | -B*D-CARRYIN
      | -B*D-P
      | -B*D-P-CARRYIN
      | -BCIN*(A+D)
      | -BCIN*(A+D)-C
      | -BCIN*(A+D)-C-CARRYIN
      | -BCIN*(A+D)-CARRYIN
      | -BCIN*(A+D)-P
      | -BCIN*(A+D)-P-CARRYIN
      | -BCIN*(ACIN+D)
      | -BCIN*(ACIN+D)-C
      | -BCIN*(ACIN+D)-C-CARRYIN
      | -BCIN*(ACIN+D)-CARRYIN
      | -BCIN*(ACIN+D)-P
      | -BCIN*(ACIN+D)-P-CARRYIN
      | -BCIN*(D+A)
      | -BCIN*(D+A)-C
      | -BCIN*(D+A)-C-CARRYIN
      | -BCIN*(D+A)-CARRYIN
      | -BCIN*(D+A)-P
      | -BCIN*(D+A)-P-CARRYIN
      | -BCIN*(D+ACIN)
      | -BCIN*(D+ACIN)-C
      | -BCIN*(D+ACIN)-C-CARRYIN
      | -BCIN*(D+ACIN)-CARRYIN
      | -BCIN*(D+ACIN)-P
      | -BCIN*(D+ACIN)-P-CARRYIN
      | -BCIN*(D-A)
      | -BCIN*(D-A)-C
      | -BCIN*(D-A)-C-CARRYIN
      | -BCIN*(D-A)-CARRYIN
      | -BCIN*(D-A)-P
      | -BCIN*(D-A)-P-CARRYIN
      | -BCIN*(D-ACIN)
      | -BCIN*(D-ACIN)-C
      | -BCIN*(D-ACIN)-C-CARRYIN
      | -BCIN*(D-ACIN)-CARRYIN
      | -BCIN*(D-ACIN)-P
      | -BCIN*(D-ACIN)-P-CARRYIN
      | -BCIN*A
      | -BCIN*A-C
      | -BCIN*A-C-CARRYIN
      | -BCIN*A-CARRYIN
      | -BCIN*A-P
      | -BCIN*A-P-CARRYIN
      | -BCIN*ACIN
      | -BCIN*ACIN-C
      | -BCIN*ACIN-C-CARRYIN
      | -BCIN*ACIN-CARRYIN
      | -BCIN*ACIN-P
      | -BCIN*ACIN-P-CARRYIN
      | -BCIN*D
      | -BCIN*D-C
      | -BCIN*D-C-CARRYIN
      | -BCIN*D-CARRYIN
      | -BCIN*D-P
      | -BCIN*D-P-CARRYIN
      | -C
      | -C+(A+D)
      | -C+(A+D)*(A+D)
      | -C+(A+D)*(A+D)+C
      | -C+(A+D)*(A+D)+P
      | -C+(A+D)*B
      | -C+(A+D)*B+C
      | -C+(A+D)*B+P
      | -C+(A+D)*BCIN
      | -C+(A+D)*BCIN+C
      | -C+(A+D)*BCIN+P
      | -C+(A+D)+C
      | -C+(A+D)+P
      | -C+(ACIN+D)
      | -C+(ACIN+D)*(ACIN+D)
      | -C+(ACIN+D)*(ACIN+D)+C
      | -C+(ACIN+D)*(ACIN+D)+P
      | -C+(ACIN+D)*B
      | -C+(ACIN+D)*B+C
      | -C+(ACIN+D)*B+P
      | -C+(ACIN+D)*BCIN
      | -C+(ACIN+D)*BCIN+C
      | -C+(ACIN+D)*BCIN+P
      | -C+(ACIN+D)+C
      | -C+(ACIN+D)+P
      | -C+(D+A)
      | -C+(D+A)*(D+A)
      | -C+(D+A)*(D+A)+C
      | -C+(D+A)*(D+A)+P
      | -C+(D+A)*B
      | -C+(D+A)*B+C
      | -C+(D+A)*B+P
      | -C+(D+A)*BCIN
      | -C+(D+A)*BCIN+C
      | -C+(D+A)*BCIN+P
      | -C+(D+A)+C
      | -C+(D+A)+P
      | -C+(D+ACIN)
      | -C+(D+ACIN)*(D+ACIN)
      | -C+(D+ACIN)*(D+ACIN)+C
      | -C+(D+ACIN)*(D+ACIN)+P
      | -C+(D+ACIN)*B
      | -C+(D+ACIN)*B+C
      | -C+(D+ACIN)*B+P
      | -C+(D+ACIN)*BCIN
      | -C+(D+ACIN)*BCIN+C
      | -C+(D+ACIN)*BCIN+P
      | -C+(D+ACIN)+C
      | -C+(D+ACIN)+P
      | -C+(D-A)
      | -C+(D-A)*(D-A)
      | -C+(D-A)*(D-A)+C
      | -C+(D-A)*(D-A)+P
      | -C+(D-A)*B
      | -C+(D-A)*B+C
      | -C+(D-A)*B+P
      | -C+(D-A)*BCIN
      | -C+(D-A)*BCIN+C
      | -C+(D-A)*BCIN+P
      | -C+(D-A)+C
      | -C+(D-A)+P
      | -C+(D-ACIN)
      | -C+(D-ACIN)*(D-ACIN)
      | -C+(D-ACIN)*(D-ACIN)+C
      | -C+(D-ACIN)*(D-ACIN)+P
      | -C+(D-ACIN)*B
      | -C+(D-ACIN)*B+C
      | -C+(D-ACIN)*B+P
      | -C+(D-ACIN)*BCIN
      | -C+(D-ACIN)*BCIN+C
      | -C+(D-ACIN)*BCIN+P
      | -C+(D-ACIN)+C
      | -C+(D-ACIN)+P
      | -C+A
      | -C+A*A
      | -C+A*A+C
      | -C+A*A+P
      | -C+A*B
      | -C+A*B+C
      | -C+A*B+P
      | -C+A*BCIN
      | -C+A*BCIN+C
      | -C+A*BCIN+P
      | -C+A+C
      | -C+A+P
      | -C+ACIN
      | -C+ACIN*ACIN
      | -C+ACIN*ACIN+C
      | -C+ACIN*ACIN+P
      | -C+ACIN*B
      | -C+ACIN*B+C
      | -C+ACIN*B+P
      | -C+ACIN*BCIN
      | -C+ACIN*BCIN+C
      | -C+ACIN*BCIN+P
      | -C+ACIN+C
      | -C+ACIN+P
      | -C+B*(A+D)
      | -C+B*(A+D)+C
      | -C+B*(A+D)+P
      | -C+B*(ACIN+D)
      | -C+B*(ACIN+D)+C
      | -C+B*(ACIN+D)+P
      | -C+B*(D+A)
      | -C+B*(D+A)+C
      | -C+B*(D+A)+P
      | -C+B*(D+ACIN)
      | -C+B*(D+ACIN)+C
      | -C+B*(D+ACIN)+P
      | -C+B*(D-A)
      | -C+B*(D-A)+C
      | -C+B*(D-A)+P
      | -C+B*(D-ACIN)
      | -C+B*(D-ACIN)+C
      | -C+B*(D-ACIN)+P
      | -C+B*A
      | -C+B*A+C
      | -C+B*A+P
      | -C+B*ACIN
      | -C+B*ACIN+C
      | -C+B*ACIN+P
      | -C+B*D
      | -C+B*D+C
      | -C+B*D+P
      | -C+BCIN*(A+D)
      | -C+BCIN*(A+D)+C
      | -C+BCIN*(A+D)+P
      | -C+BCIN*(ACIN+D)
      | -C+BCIN*(ACIN+D)+C
      | -C+BCIN*(ACIN+D)+P
      | -C+BCIN*(D+A)
      | -C+BCIN*(D+A)+C
      | -C+BCIN*(D+A)+P
      | -C+BCIN*(D+ACIN)
      | -C+BCIN*(D+ACIN)+C
      | -C+BCIN*(D+ACIN)+P
      | -C+BCIN*(D-A)
      | -C+BCIN*(D-A)+C
      | -C+BCIN*(D-A)+P
      | -C+BCIN*(D-ACIN)
      | -C+BCIN*(D-ACIN)+C
      | -C+BCIN*(D-ACIN)+P
      | -C+BCIN*A
      | -C+BCIN*A+C
      | -C+BCIN*A+P
      | -C+BCIN*ACIN
      | -C+BCIN*ACIN+C
      | -C+BCIN*ACIN+P
      | -C+BCIN*D
      | -C+BCIN*D+C
      | -C+BCIN*D+P
      | -C+C
      | -C+C+(A+D)
      | -C+C+(A+D)*(A+D)
      | -C+C+(A+D)*B
      | -C+C+(A+D)*BCIN
      | -C+C+(ACIN+D)
      | -C+C+(ACIN+D)*(ACIN+D)
      | -C+C+(ACIN+D)*B
      | -C+C+(ACIN+D)*BCIN
      | -C+C+(D+A)
      | -C+C+(D+A)*(D+A)
      | -C+C+(D+A)*B
      | -C+C+(D+A)*BCIN
      | -C+C+(D+ACIN)
      | -C+C+(D+ACIN)*(D+ACIN)
      | -C+C+(D+ACIN)*B
      | -C+C+(D+ACIN)*BCIN
      | -C+C+(D-A)
      | -C+C+(D-A)*(D-A)
      | -C+C+(D-A)*B
      | -C+C+(D-A)*BCIN
      | -C+C+(D-ACIN)
      | -C+C+(D-ACIN)*(D-ACIN)
      | -C+C+(D-ACIN)*B
      | -C+C+(D-ACIN)*BCIN
      | -C+C+A
      | -C+C+A*A
      | -C+C+A*B
      | -C+C+A*BCIN
      | -C+C+ACIN
      | -C+C+ACIN*ACIN
      | -C+C+ACIN*B
      | -C+C+ACIN*BCIN
      | -C+C+B*(A+D)
      | -C+C+B*(ACIN+D)
      | -C+C+B*(D+A)
      | -C+C+B*(D+ACIN)
      | -C+C+B*(D-A)
      | -C+C+B*(D-ACIN)
      | -C+C+B*A
      | -C+C+B*ACIN
      | -C+C+B*D
      | -C+C+BCIN*(A+D)
      | -C+C+BCIN*(ACIN+D)
      | -C+C+BCIN*(D+A)
      | -C+C+BCIN*(D+ACIN)
      | -C+C+BCIN*(D-A)
      | -C+C+BCIN*(D-ACIN)
      | -C+C+BCIN*A
      | -C+C+BCIN*ACIN
      | -C+C+BCIN*D
      | -C+C+C
      | -C+C+C+CONCAT
      | -C+C+C+P
      | -C+C+CONCAT
      | -C+C+CONCAT+C
      | -C+C+CONCAT+P
      | -C+C+D
      | -C+C+D*B
      | -C+C+D*BCIN
      | -C+C+D*D
      | -C+C+P
      | -C+C+P+C
      | -C+C+P+CONCAT
      | -C+C+P+P
      | -C+CONCAT
      | -C+CONCAT+C
      | -C+CONCAT+C+C
      | -C+CONCAT+C+P
      | -C+CONCAT+P
      | -C+CONCAT+P+C
      | -C+D
      | -C+D*B
      | -C+D*B+C
      | -C+D*B+P
      | -C+D*BCIN
      | -C+D*BCIN+C
      | -C+D*BCIN+P
      | -C+D*D
      | -C+D*D+C
      | -C+D*D+P
      | -C+D+C
      | -C+D+P
      | -C+P
      | -C+P+(A+D)
      | -C+P+(A+D)*(A+D)
      | -C+P+(A+D)*B
      | -C+P+(A+D)*BCIN
      | -C+P+(ACIN+D)
      | -C+P+(ACIN+D)*(ACIN+D)
      | -C+P+(ACIN+D)*B
      | -C+P+(ACIN+D)*BCIN
      | -C+P+(D+A)
      | -C+P+(D+A)*(D+A)
      | -C+P+(D+A)*B
      | -C+P+(D+A)*BCIN
      | -C+P+(D+ACIN)
      | -C+P+(D+ACIN)*(D+ACIN)
      | -C+P+(D+ACIN)*B
      | -C+P+(D+ACIN)*BCIN
      | -C+P+(D-A)
      | -C+P+(D-A)*(D-A)
      | -C+P+(D-A)*B
      | -C+P+(D-A)*BCIN
      | -C+P+(D-ACIN)
      | -C+P+(D-ACIN)*(D-ACIN)
      | -C+P+(D-ACIN)*B
      | -C+P+(D-ACIN)*BCIN
      | -C+P+A
      | -C+P+A*A
      | -C+P+A*B
      | -C+P+A*BCIN
      | -C+P+ACIN
      | -C+P+ACIN*ACIN
      | -C+P+ACIN*B
      | -C+P+ACIN*BCIN
      | -C+P+B*(A+D)
      | -C+P+B*(ACIN+D)
      | -C+P+B*(D+A)
      | -C+P+B*(D+ACIN)
      | -C+P+B*(D-A)
      | -C+P+B*(D-ACIN)
      | -C+P+B*A
      | -C+P+B*ACIN
      | -C+P+B*D
      | -C+P+BCIN*(A+D)
      | -C+P+BCIN*(ACIN+D)
      | -C+P+BCIN*(D+A)
      | -C+P+BCIN*(D+ACIN)
      | -C+P+BCIN*(D-A)
      | -C+P+BCIN*(D-ACIN)
      | -C+P+BCIN*A
      | -C+P+BCIN*ACIN
      | -C+P+BCIN*D
      | -C+P+C
      | -C+P+C+C
      | -C+P+C+CONCAT
      | -C+P+C+P
      | -C+P+CONCAT
      | -C+P+CONCAT+C
      | -C+P+D
      | -C+P+D*B
      | -C+P+D*BCIN
      | -C+P+D*D
      | -C+P+P
      | -C+P+P+C
      | -C-((A+D))
      | -C-((A+D)*(A+D))
      | -C-((A+D)*B)
      | -C-((A+D)*BCIN)
      | -C-((ACIN+D))
      | -C-((ACIN+D)*(ACIN+D))
      | -C-((ACIN+D)*B)
      | -C-((ACIN+D)*BCIN)
      | -C-((D+A))
      | -C-((D+A)*(D+A))
      | -C-((D+A)*B)
      | -C-((D+A)*BCIN)
      | -C-((D+ACIN))
      | -C-((D+ACIN)*(D+ACIN))
      | -C-((D+ACIN)*B)
      | -C-((D+ACIN)*BCIN)
      | -C-((D-A))
      | -C-((D-A)*(D-A))
      | -C-((D-A)*B)
      | -C-((D-A)*BCIN)
      | -C-((D-ACIN))
      | -C-((D-ACIN)*(D-ACIN))
      | -C-((D-ACIN)*B)
      | -C-((D-ACIN)*BCIN)
      | -C-(A)
      | -C-(A*A)
      | -C-(A*B)
      | -C-(A*BCIN)
      | -C-(A+D)
      | -C-(A+D)*(A+D)
      | -C-(A+D)*(A+D)-CARRYIN
      | -C-(A+D)*B
      | -C-(A+D)*B-CARRYIN
      | -C-(A+D)*BCIN
      | -C-(A+D)*BCIN-CARRYIN
      | -C-(A+D)-CARRYIN
      | -C-(ACIN)
      | -C-(ACIN*ACIN)
      | -C-(ACIN*B)
      | -C-(ACIN*BCIN)
      | -C-(ACIN+D)
      | -C-(ACIN+D)*(ACIN+D)
      | -C-(ACIN+D)*(ACIN+D)-CARRYIN
      | -C-(ACIN+D)*B
      | -C-(ACIN+D)*B-CARRYIN
      | -C-(ACIN+D)*BCIN
      | -C-(ACIN+D)*BCIN-CARRYIN
      | -C-(ACIN+D)-CARRYIN
      | -C-(B*(A+D))
      | -C-(B*(ACIN+D))
      | -C-(B*(D+A))
      | -C-(B*(D+ACIN))
      | -C-(B*(D-A))
      | -C-(B*(D-ACIN))
      | -C-(B*A)
      | -C-(B*ACIN)
      | -C-(B*D)
      | -C-(BCIN*(A+D))
      | -C-(BCIN*(ACIN+D))
      | -C-(BCIN*(D+A))
      | -C-(BCIN*(D+ACIN))
      | -C-(BCIN*(D-A))
      | -C-(BCIN*(D-ACIN))
      | -C-(BCIN*A)
      | -C-(BCIN*ACIN)
      | -C-(BCIN*D)
      | -C-(D)
      | -C-(D*B)
      | -C-(D*BCIN)
      | -C-(D*D)
      | -C-(D+A)
      | -C-(D+A)*(D+A)
      | -C-(D+A)*(D+A)-CARRYIN
      | -C-(D+A)*B
      | -C-(D+A)*B-CARRYIN
      | -C-(D+A)*BCIN
      | -C-(D+A)*BCIN-CARRYIN
      | -C-(D+A)-CARRYIN
      | -C-(D+ACIN)
      | -C-(D+ACIN)*(D+ACIN)
      | -C-(D+ACIN)*(D+ACIN)-CARRYIN
      | -C-(D+ACIN)*B
      | -C-(D+ACIN)*B-CARRYIN
      | -C-(D+ACIN)*BCIN
      | -C-(D+ACIN)*BCIN-CARRYIN
      | -C-(D+ACIN)-CARRYIN
      | -C-(D-A)
      | -C-(D-A)*(D-A)
      | -C-(D-A)*(D-A)-CARRYIN
      | -C-(D-A)*B
      | -C-(D-A)*B-CARRYIN
      | -C-(D-A)*BCIN
      | -C-(D-A)*BCIN-CARRYIN
      | -C-(D-A)-CARRYIN
      | -C-(D-ACIN)
      | -C-(D-ACIN)*(D-ACIN)
      | -C-(D-ACIN)*(D-ACIN)-CARRYIN
      | -C-(D-ACIN)*B
      | -C-(D-ACIN)*B-CARRYIN
      | -C-(D-ACIN)*BCIN
      | -C-(D-ACIN)*BCIN-CARRYIN
      | -C-(D-ACIN)-CARRYIN
      | -C-A
      | -C-A*A
      | -C-A*A-CARRYIN
      | -C-A*B
      | -C-A*B-CARRYIN
      | -C-A*BCIN
      | -C-A*BCIN-CARRYIN
      | -C-A-CARRYIN
      | -C-ACIN
      | -C-ACIN*ACIN
      | -C-ACIN*ACIN-CARRYIN
      | -C-ACIN*B
      | -C-ACIN*B-CARRYIN
      | -C-ACIN*BCIN
      | -C-ACIN*BCIN-CARRYIN
      | -C-ACIN-CARRYIN
      | -C-B*(A+D)
      | -C-B*(A+D)-CARRYIN
      | -C-B*(ACIN+D)
      | -C-B*(ACIN+D)-CARRYIN
      | -C-B*(D+A)
      | -C-B*(D+A)-CARRYIN
      | -C-B*(D+ACIN)
      | -C-B*(D+ACIN)-CARRYIN
      | -C-B*(D-A)
      | -C-B*(D-A)-CARRYIN
      | -C-B*(D-ACIN)
      | -C-B*(D-ACIN)-CARRYIN
      | -C-B*A
      | -C-B*A-CARRYIN
      | -C-B*ACIN
      | -C-B*ACIN-CARRYIN
      | -C-B*D
      | -C-B*D-CARRYIN
      | -C-BCIN*(A+D)
      | -C-BCIN*(A+D)-CARRYIN
      | -C-BCIN*(ACIN+D)
      | -C-BCIN*(ACIN+D)-CARRYIN
      | -C-BCIN*(D+A)
      | -C-BCIN*(D+A)-CARRYIN
      | -C-BCIN*(D+ACIN)
      | -C-BCIN*(D+ACIN)-CARRYIN
      | -C-BCIN*(D-A)
      | -C-BCIN*(D-A)-CARRYIN